simulink model "TosAeb"  (R2021a)
settings: Solver=FixedStepDiscrete  StartTime=0.0  StopTime=inf  FixedStep=0.01  RelTol=1e-3
undefined symbols (not defined in the file or in its folder): ACCModSts, AccSts1, AEB_ObjectMovingOrientation, AEB_ObjectMovingState, CN_BTCActive, CN_CurrentGearR, CN_CurrentGearR_DHT, CN_PTCActive, CN_VDCActive, CN_VSC_EspOff, CN_VSC_FCW_AEB_Error, CN_VSC_FCW_AEB_NoDispay, CN_VSC_FCW_AEB_PerfmDgdtn, CN_VSC_FCW_AEB_TempUnAvlbl, CN_VSC_VehStandstill, CNaccelAvoidMax, CNaccelAvoidMin, CNbrakePedalPress, CNcomfortStrLeftAccelMax, CNcomfortStrLeftAccelMin, CNcomfortStrRightAccelMax, CNcomfortStrRightAccelMin, CNDrvDoorOpen, CNDrvModeEco, CNDrvModeNorm, CNDrvModeOther, CNDrvModeSport, CNDrvSBRreminder, CNNull, CNobjInvalidID, CNPassDoorOpen, CNPassSBRreminder, CNPEBSAccelNegInf, CNPEBSAccelNull, CNPEBSTimeInf, CNPEBSTimeNull, CNSTOPMAX, CNSTOPNULL, CNsubjWidth, CNSwtOFF, CNSwtON, CNTTCMAX, CNTTCNULL, CNVehConfigAEBCycNone, CNVehConfigAEBNone, CNVehConfigAEBPedNone, CNVehConfigFCWNone, CNVehConfigJANone, CollisionSeverity, FALSE, FCWSnvty, JACycObjCalcInfoDefaultParams, JAPedObjCalcInfoDefaultParams, JAVehObjCalcInfoDefaultParams, latCycObjCalcInfoDefaultParams, latPedObjCalcInfoDefaultParams, longObjCalcInfoDefaultParams, ModSts, movingStatus, ObjectType ... (+1112)
libraries: simulink x3031, AEBSFunctionLib x50, Ta_Library x33, AEBSStateMachineLib x23, Mylibrary x12, simulink_extras x7, ObjSelect_lib x3, dspstat3 x2
(part 2 of 102)

== system TosAeb/FcwAebJa_Step_sys/ALG_AEBSystem ==
  TOS_AEB_LONOBJ : Inport  OutDataTypeStr=Bus: Object_Struct  BusOutputAsStruct=on
  TOS_AEB_LATOBJ : Inport  Port=2  OutDataTypeStr=Bus: Object_Struct  PortDimensions=10  Interpolate=off  BusOutputAsStruct=on
  TOS_AEB_JAOBJ : Inport  Port=3  OutDataTypeStr=Bus: Object_Struct  PortDimensions=32  Interpolate=off  BusOutputAsStruct=on
  vxvRefMs : Inport  Port=4  OutDataTypeStr=single  PortDimensions=1
  axvRefMs2 : Inport  Port=5  OutDataTypeStr=single  PortDimensions=1
  ayvRefMs2 : Inport  Port=6  OutDataTypeStr=single  PortDimensions=1
  kapTraj : Inport  Port=7  OutDataTypeStr=single  PortDimensions=1
  psiDtOpt : Inport  Port=8  OutDataTypeStr=single  PortDimensions=1
  ALG_VCAN_MSG : Inport  Port=9  OutDataTypeStr=Bus: VehicleInfo_Struct  PortDimensions=1  BusOutputAsStruct=on
  ALG_AEBController : Subsystem  TreatAsAtomicUnit=on
  ALG_AEBInput : Subsystem  TreatAsAtomicUnit=on
  ALG_AEBOutput : Subsystem  TreatAsAtomicUnit=on
  Signal Conversion{3..7} (5 alike) : SignalConversion  OverrideOpt=off
  AEB_BRK_INFO : Outport  OutDataTypeStr=Bus: AEB_BRK_INFO_BUS  PortDimensions=1
  AEB_DVR_INFO : Outport  Port=2  OutDataTypeStr=Bus: AEB_DVR_INFO_BUS  PortDimensions=1
  AEB_IPHUT_INFO : Outport  Port=3  OutDataTypeStr=Bus: AEB_IPHUT_INFO_BUS  PortDimensions=1
  AEB_EDR_LONOBJ_INFO : Outport  Port=4  OutDataTypeStr=Bus: AEB_EDR_LONOBJ_INFO_BUS  PortDimensions=1
  AEB_EDR_LATPED_INFO : Outport  Port=5  OutDataTypeStr=Bus: AEB_EDR_LATPED_INFO_BUS  PortDimensions=1
  AEB_EDR_LATCYC_INFO : Outport  Port=6  OutDataTypeStr=Bus: AEB_EDR_LATCYC_INFO_BUS  PortDimensions=1
  AEB_EDR_JAPED_INFO : Outport  Port=7  OutDataTypeStr=Bus: AEB_EDR_JAPED_INFO_BUS  PortDimensions=1
  AEB_EDR_JAVEH_INFO : Outport  Port=8  OutDataTypeStr=Bus: AEB_EDR_JAVEH_INFO_BUS  PortDimensions=1
  AEB_FUNC_STS_INFO : Outport  Port=9  OutDataTypeStr=Bus: AEB_FUNC_STS_INFO_BUS
  wires:
  ALG_AEBInput/1(diaManageInfo) -> ALG_AEBController/1(diaManageInfo)
  ALG_AEBInput/2(vehCanInfo) -> ALG_AEBController/2(vehCanInfo)
  ALG_AEBInput/3(vehConfigInfo) -> ALG_AEBController/3(vehConfigInfo)
  ALG_AEBInput/4(longObjInfo) -> ALG_AEBController/4(longObjInfo)
  ALG_AEBInput/5(subjInfo) -> ALG_AEBController/5(subjInfo)
  ALG_AEBInput/6(ACCState) -> ALG_AEBController/6(ACCState)
  ALG_AEBInput/7(LCK_Active) -> ALG_AEBController/7(LCK_Active)
  ALG_AEBInput/8(latObjInfo) -> ALG_AEBController/8(latObjInfo)
  ALG_AEBInput/9(JaObjInfo) -> ALG_AEBController/9(JaObjInfo)
  ALG_AEBController/1(DiaManageInfo) -> ALG_AEBOutput/1(DiaManageInfo)
  ALG_AEBController/2(VehCanInfoOut) -> ALG_AEBOutput/2(VehCanInfo)
  ALG_AEBController/3(vehConfigInfoOut) -> ALG_AEBOutput/3(vehConfigInfoOut)
  ALG_AEBController/4(latPedCalcInfo) -> ALG_AEBOutput/4(latPedCalcInfo)
  ALG_AEBController/5(latCycCalcInfo) -> ALG_AEBOutput/5(latCycCalcInfo)
  ALG_AEBController/6(AEBSFuncOut) -> ALG_AEBOutput/6(AEBSFuncOut)
  ALG_AEBController/7(vehStateCalcInfo) -> ALG_AEBOutput/7(vehStateCalcInfo)
  ALG_AEBController/8(longObjCalcInfo) -> ALG_AEBOutput/8(longObjCalcInfo)
  ALG_AEBController/9(AEBModStsFuncImpArb) -> ALG_AEBOutput/9(AEBModStsFuncImpArb)
  ALG_AEBController/10(AEBModRawSts) -> ALG_AEBOutput/10(AEBModRawSts)
  ALG_AEBController/11(latPedTgtCalcInfo) -> ALG_AEBOutput/11(latPedTgtCalcInfo)
  ALG_AEBController/12(latCycTgtCalcInfo) -> ALG_AEBOutput/12(latCycTgtCalcInfo)
  ALG_AEBController/13(AEBJAPedTgtCalcInfo) -> ALG_AEBOutput/13(AEBJAPedTgtCalcInfo)
  ALG_AEBController/14(JAVehTgtCalcInfo) -> ALG_AEBOutput/14(JAVehTgtCalcInfo)
  ALG_AEBOutput/7(AEB_EDR_JAPED_INFO) -> AEB_EDR_JAPED_INFO/1
  ALG_AEBOutput/8(AEB_EDR_JAVEH_INFO) -> AEB_EDR_JAVEH_INFO/1
  ALG_AEBOutput/9(AEB_FUNC_STS_INFO) -> AEB_FUNC_STS_INFO/1
  ALG_AEBOutput/3(AEB_IPHUT_INFO) -> AEB_IPHUT_INFO/1
  ALG_AEBOutput/6(AEB_EDR_LATCYC_INFO) -> AEB_EDR_LATCYC_INFO/1
  ALG_AEBOutput/4(AEB_EDR_LONOBJ_INFO) -> AEB_EDR_LONOBJ_INFO/1
  ALG_AEBOutput/5(AEB_EDR_LATPED_INFO) -> AEB_EDR_LATPED_INFO/1
  ALG_AEBOutput/2(AEB_DVR_INFO) -> AEB_DVR_INFO/1
  ALG_AEBOutput/1(AEB_BRK_INFO) -> AEB_BRK_INFO/1
  TOS_AEB_JAOBJ/1 -> ALG_AEBInput/3(TOS_AEB_JAOBJ)  "AEB_TOS_AEB_JAOBJ"
  Signal Conversion3/1 -> ALG_AEBInput/4(vxvRefMs)  "AEB_vxvRefMs"
  ayvRefMs2/1 -> Signal Conversion5/1
  Signal Conversion5/1 -> ALG_AEBInput/6(ayvRefMs2)  "AEB_ayvRefMs2"
  TOS_AEB_LONOBJ/1 -> ALG_AEBInput/1(TOS_AEB_LONOBJ)  "AEB_TOS_AEB_LONOBJ"
  TOS_AEB_LATOBJ/1 -> ALG_AEBInput/2(TOS_AEB_LATOBJ)  "AEB_TOS_AEB_LATOBJ"
  Signal Conversion6/1 -> ALG_AEBInput/7(kapTraj)  "AEB_kapTraj"
  kapTraj/1 -> Signal Conversion6/1
  Signal Conversion7/1 -> ALG_AEBInput/8(psiDtOpt)  "AEB_psiDtOpt"
  psiDtOpt/1 -> Signal Conversion7/1
  Signal Conversion4/1 -> ALG_AEBInput/5(axvRefMs2)  "AEB_axvRefMs2"
  axvRefMs2/1 -> Signal Conversion4/1
  vxvRefMs/1 -> Signal Conversion3/1
  ALG_VCAN_MSG/1 -> ALG_AEBInput/9(ALG_VCAN_MSG)
  ALG_AEBController/15(FCWSnvtyALGInfo) -> ALG_AEBOutput/15(FCWSnvtyALGInfo)

== system TosAeb/AEB_Objsel/TA_InfoRebuild/TAObjInfoCalc ==
  Fus_TargetBus : Inport
  Road_Info : Inport  Port=2
  Bus Selector : BusSelector  OutputSignals=Cam_ObsID_u8,Cam_laneLeftPortion,Cam_laneAssociateConf,Cam_laneRightPortion,Cam_laneLeftConf,Cam_laneRightConf,Cam_objIntention,Cam_objIntentionProb,Cam_laneAssign,Cam_ObsPosX_s,Cam_ObsPosY_s,Cam_ObsAccY_s,Cam_ObsAccX_s,Cam_ObsVelY_s,Cam_ObsVelX_s,Cam_ObsID_u8,Cam_ObsValid_u8
  For Each Subsystem : Subsystem  TreatAsAtomicUnit=on
  Signal_Rebuild : Subsystem
  Objrebuild_Struct : Outport
  wires:
  Fus_TargetBus/1 -> Bus Selector/1
  Bus Selector/1 -> For Each Subsystem/1(IDForEach)  "<Cam_ObsID_u8>"
  Bus Selector/15 -> For Each Subsystem/15(Cam_ObsVelX_s)  "<Cam_ObsVelX_s>"
  Bus Selector/14 -> For Each Subsystem/14(Cam_ObsVelY_s)  "<Cam_ObsVelY_s>"
  Bus Selector/13 -> For Each Subsystem/13(Cam_ObsAccX_s)  "<Cam_ObsAccX_s>"
  Bus Selector/12 -> For Each Subsystem/12(Cam_ObsAccY_s)  "<Cam_ObsAccY_s>"
  Bus Selector/11 -> For Each Subsystem/11(Cam_ObsPosY_s)  "<Cam_ObsPosY_s>"
  Bus Selector/10 -> For Each Subsystem/10(Cam_ObsPosX_s)  "<Cam_ObsPosX_s>"
  Bus Selector/9 -> For Each Subsystem/9(Cam_laneAssign)  "<Cam_laneAssign>"
  Bus Selector/8 -> For Each Subsystem/8(Cam_objIntentionProb)  "<Cam_objIntentionProb>"
  Bus Selector/7 -> For Each Subsystem/7(Cam_objIntention)  "<Cam_objIntention>"
  Bus Selector/6 -> For Each Subsystem/6(Cam_laneRightConf)  "<Cam_laneRightConf>"
  Bus Selector/5 -> For Each Subsystem/5(Cam_laneLeftConf)  "<Cam_laneLeftConf>"
  Bus Selector/4 -> For Each Subsystem/4(Cam_laneRightPortion)  "<Cam_laneRightPortion>"
  Bus Selector/3 -> For Each Subsystem/3(Cam_laneAssociateConf)  "<Cam_laneAssociateConf>"
  Bus Selector/2 -> For Each Subsystem/2(Cam_laneLeftPortion)  "<Cam_laneLeftPortion>"
  Road_Info/1 -> For Each Subsystem/18(Road_Info)
  For Each Subsystem/6(ayRel) -> Signal_Rebuild/6(ayRel)
  For Each Subsystem/5(axRel) -> Signal_Rebuild/5(axRel)
  For Each Subsystem/7(axRelStd) -> Signal_Rebuild/7(axRelStd)
  For Each Subsystem/4(vyRelStd) -> Signal_Rebuild/4(vyRelStd)
  For Each Subsystem/8(ayRelStd) -> Signal_Rebuild/8(ayRelStd)
  For Each Subsystem/3(vxRelStd) -> Signal_Rebuild/3(vxRelStd)
  For Each Subsystem/9(azimuthAngle) -> Signal_Rebuild/9(azimuthAngle)
  For Each Subsystem/2(vyRel) -> Signal_Rebuild/2(vyRel)
  For Each Subsystem/10(azimuthAngleStd) -> Signal_Rebuild/10(azimuthAngleStd)
  For Each Subsystem/1(vxRel) -> Signal_Rebuild/1(vxRel)
  For Each Subsystem/11(objLatDist2LaneCenter) -> Signal_Rebuild/11(objLatDist2LaneCenter)
  For Each Subsystem/12(objLatDist2EgoCenter) -> Signal_Rebuild/12(objLatDist2EgoCenter)
  For Each Subsystem/13(objPosition) -> Signal_Rebuild/13(objPosition)
  Signal_Rebuild/1(Objrebuild_Struct) -> Objrebuild_Struct/1
  Bus Selector/16 -> For Each Subsystem/16(Cam_ObsID_u8)  "<Cam_ObsID_u8>"
  Bus Selector/17 -> For Each Subsystem/17(Cam_ObsValid_u8)  "<Cam_ObsValid_u8>"

== system TosAeb/AEB_Objsel/TA_Processor/AEB_ObjSelect ==
  VehSelfData : Inport
  TA_ObjSumInfo : Inport  Port=2
  PathEstimn : Inport  Port=3
  AccTgtIdn : Inport  Port=4
  TargetInfoCheck : Subsystem
  TargetSelect : Subsystem
  Long_ObjSortNum : Outport
  Long_ObjID : Outport  Port=2
  Lat_ObjSortNum : Outport  Port=3
  Lat_ObjID : Outport  Port=4
  Ta_Info : Outport  Port=5
  Long_TTC : Outport  Port=6
  Lat_TTC : Outport  Port=7
  JA_TTC : Outport  Port=8
  wires:
  TargetInfoCheck/11(JA_TTRAssign) -> TargetSelect/11(JA_TTRAssign)
  TA_ObjSumInfo/1 -> TargetInfoCheck/1(TA_ObjSumInfo)
  TargetInfoCheck/1(TA_ObjSumInfo_P) -> TargetSelect/1(TA_ObjInfo)
  VehSelfData/1 -> TargetInfoCheck/2(VehSelfData)
  TargetInfoCheck/2(VehSelfData_P) -> TargetSelect/2(VehSelfData)
  PathEstimn/1 -> TargetInfoCheck/3(PathEstimn)
  AccTgtIdn/1 -> TargetInfoCheck/4(AccTgtIdn)
  TargetSelect/5(Ta_Info) -> Ta_Info/1
  TargetInfoCheck/3(AccTgtIdn_P) -> TargetSelect/3(AccTgtIdn)
  TargetInfoCheck/5(Obj_Coef) -> TargetSelect/5(Obj_Coef)
  TargetInfoCheck/4(Boudingbox_Calculate) -> TargetSelect/4(Boudingbox_Calculate)
  TargetInfoCheck/9(Lat_TTR) -> TargetSelect/9(Lat_TTR)
  TargetInfoCheck/6(TTI) -> TargetSelect/6(TTI)
  TargetSelect/1(Long_ObjSortNum) -> Long_ObjSortNum/1
  TargetInfoCheck/7(LatObj_Valid) -> TargetSelect/7(LatObj_Valid)
  TargetInfoCheck/10(Overlap_Flg) -> TargetSelect/10(Overlap_Flg)
  TargetInfoCheck/8(Lat_ObjIntersect) -> TargetSelect/8(Lat_ObjIntersect)
  TargetSelect/3(Lat_ObjSortNum) -> Lat_ObjSortNum/1
  TargetSelect/2(Long_ObjID) -> Long_ObjID/1
  TargetSelect/7(Lat_TTC) -> Lat_TTC/1
  TargetSelect/4(Lat_ObjID) -> Lat_ObjID/1
  TargetSelect/6(Long_TTC) -> Long_TTC/1
  TargetSelect/8(JA_TTC) -> JA_TTC/1

== system TosAeb/AEB_Objsel/TA_Processor/PreProcessor ==
  VehSelfIn : Inport
  ACC_Infout : Inport  Port=2
  Fus_TargetBus : Inport  Port=3
  ExtractAccTargetIdIfAccActive : Subsystem
  ObjectPrecessor : Subsystem
  VehiclePreProcessor : Subsystem
  VehSelfData : Outport
  TA_ObjSumInfo : Outport  Port=2
  PathEstimn : Outport  Port=3
  AccTgtIdn : Outport  Port=4
  wires:
  Fus_TargetBus/1 -> ObjectPrecessor/2(Fus_TargetBus)
  VehSelfIn/1 -> ObjectPrecessor/1(VehSelfIn), VehiclePreProcessor/1(VehSelfIn)
  ObjectPrecessor/1(TA_ObjInfo) -> TA_ObjSumInfo/1
  ExtractAccTargetIdIfAccActive/1(AccTgtIdn) -> AccTgtIdn/1
  VehiclePreProcessor/2(PathEstimn) -> PathEstimn/1
  ACC_Infout/1 -> ExtractAccTargetIdIfAccActive/1(ACC_Infout)
  VehiclePreProcessor/1(VehSelfData) -> VehSelfData/1

== system TosAeb/AEB_Objsel/TA_Processor/TA_Check ==
  Long_ObjSortNum : Inport
  Long_ObjID : Inport  Port=2
  Lat_ObjSortNum : Inport  Port=3
  Lat_ObjID : Inport  Port=4
  Ta_Info : Inport  Port=5
  Long_TTC : Inport  Port=6
  Lat_TTC : Inport  Port=7
  JA_TTC : Inport  Port=8
  Signal Conversion : SignalConversion  OverrideOpt=off
  TA_LatCheck : Subsystem
  TA_LongCheck : Subsystem
  ObjLong_Bus : Outport
  ObjLat_Bus : Outport  Port=2
  TTC_JA : Outport  Port=3
  wires:
  Lat_ObjSortNum/1 -> TA_LatCheck/1(Lat_ObjSortNum)
  Lat_ObjID/1 -> TA_LatCheck/2(Lat_ObjID)
  Lat_TTC/1 -> TA_LatCheck/3(Lat_TTC)
  Long_ObjSortNum/1 -> TA_LongCheck/1(Long_ObjSortNum)
  Long_ObjID/1 -> TA_LongCheck/2(Long_ObjID)
  Ta_Info/1 -> TA_LongCheck/4(TA_Info)
  Long_TTC/1 -> TA_LongCheck/3(Long_TTC)
  TA_LongCheck/1(ObjLong_Bus) -> ObjLong_Bus/1
  JA_TTC/1 -> Signal Conversion/1
  Signal Conversion/1 -> TTC_JA/1
  TA_LatCheck/1(ObjLat_Bus) -> ObjLat_Bus/1

== system TosAeb/AEB_Objsel/TOS_AEB_Lat_Attributes/If Action Subsystem ==
  Action Port : ActionPort  ActionPortLabel=if(u1 == 0)
  Bus Creator : BusCreator  Inputs=2
  Constant{1..3,10..11,41..44,49..52} (13 alike) : Constant  Value=0  OutDataTypeStr=uint8
  Constant{4,6..9,12..36,45..48,53..56} (38 alike) : Constant  Value=0  OutDataTypeStr=single
  Constant37 : Constant  Value=0  OutDataTypeStr=uint16
  Constant39 : Constant  Value=0  OutDataTypeStr=boolean
  Constant5 : Constant  Value=0  OutDataTypeStr=boolean
  Out1 : Outport
  wires:
  Constant1/1 -> Bus Creator/1  "FusionType"
  Constant2/1 -> Bus Creator/2  "ObjectID"
  Bus Creator/1 -> Out1/1

== system TosAeb/AEB_Objsel/TOS_AEB_Lat_Attributes/If Action Subsystem1 ==
  TOS_AEB_Lat_Index : Inport
  TOS_PP_OFM_SignalVerification : Inport  Port=2
  Action Port : ActionPort  ActionPortLabel=else
  Bus Creator : BusCreator  Inputs=2
  Bus Selector2 : BusSelector  OutputSignals=objID,objType
  Selector : Selector  InputPortWidth=32  IndexOptions=Index vector (port)  Indices=[1 3]  OutputSizes=1
  Selector1 : Selector  InputPortWidth=32  IndexOptions=Index vector (port)  Indices=[1 3]  OutputSizes=1
  Out1 : Outport
  wires:
  TOS_AEB_Lat_Index/1 -> Selector1/2, Selector/2
  TOS_PP_OFM_SignalVerification/1 -> Selector1/1, Selector/1, Bus Selector2/1
  Bus Creator/1 -> Out1/1
  Selector/1 -> Bus Creator/1  "FusionType"
  Selector1/1 -> Bus Creator/2  "ObjectID"

== system TosAeb/ELK_Objsel/ELK_Left_Obj_Delay/Trigger Hold1 ==
  Trigger : Inport
  hold_time : Inport  Port=2
  Chart : Stateflow chart  TreatAsAtomicUnit=on
  Out : Outport
  wires:
  hold_time/1 -> Chart/2(hold_time)
  Trigger/1 -> Chart/1(Trigger)
  Chart/1(Out) -> Out/1

== system TosAeb/ELK_Objsel/ELK_Left_Obj_Delay1/Trigger Hold1 ==
  Trigger : Inport
  hold_time : Inport  Port=2
  Chart : Stateflow chart  TreatAsAtomicUnit=on
  Out : Outport
  wires:
  Chart/1(Out) -> Out/1
  Trigger/1 -> Chart/1(Trigger)
  hold_time/1 -> Chart/2(hold_time)

== system TosAeb/ELK_Objsel/LanesSelect/LineInfo_Process ==
  Leftline_Data : Inport
  Bus Selector : BusSelector  OutputSignals=lineSegNum,lineSegs
  Bus Selector1 : BusSelector  OutputSignals=lineSegValid,lineSegStart,lineSegEnd,lineC0,lineC1,lineC2,lineC3
  Bus Selector2 : BusSelector  OutputSignals=lineSegValid,lineSegStart,lineSegEnd,lineC0,lineC1,lineC2,lineC3
  Bus Selector3 : BusSelector  OutputSignals=lineSegValid,lineSegStart,lineSegEnd,lineC0,lineC1,lineC2,lineC3
  Line_Info : MATLAB Function  TreatAsAtomicUnit=on
  Selector18 : Selector  IndexMode=Zero-based  InputPortWidth=3  IndexOptions=Index vector (dialog)  Indices=[0]  OutputSizes=1
  Selector2 : Selector  IndexMode=Zero-based  InputPortWidth=3  IndexOptions=Index vector (dialog)  Indices=[1]  OutputSizes=1
  Selector3 : Selector  IndexMode=Zero-based  InputPortWidth=3  IndexOptions=Index vector (dialog)  Indices=[2]  OutputSizes=1
  wires:
  Leftline_Data/1 -> Bus Selector/1
  Selector3/1 -> Bus Selector3/1
  Selector18/1 -> Bus Selector1/1
  Selector2/1 -> Bus Selector2/1
  Bus Selector/2 -> Selector3/1, Selector2/1, Selector18/1  "<lineSegs>"
  Bus Selector1/1 -> Line_Info/2(seg1_valid)  "<lineSegValid>"
  Bus Selector1/2 -> Line_Info/3(seg1_Start)  "<lineSegStart>"
  Bus Selector1/3 -> Line_Info/4(seg1_end)  "<lineSegEnd>"
  Bus Selector1/4 -> Line_Info/5(seg1_C0)  "<lineC0>"
  Bus Selector1/5 -> Line_Info/6(seg1_C1)  "<lineC1>"
  Bus Selector1/6 -> Line_Info/7(seg1_C2)  "<lineC2>"
  Bus Selector1/7 -> Line_Info/8(seg1_C3)  "<lineC3>"
  Bus Selector2/1 -> Line_Info/9(seg2_valid)  "<lineSegValid>"
  Bus Selector2/2 -> Line_Info/10(seg2_Start)  "<lineSegStart>"
  Bus Selector2/3 -> Line_Info/11(seg2_end)  "<lineSegEnd>"
  Bus Selector2/4 -> Line_Info/12(seg2_C0)  "<lineC0>"
  Bus Selector2/5 -> Line_Info/13(seg2_C1)  "<lineC1>"
  Bus Selector2/6 -> Line_Info/14(seg2_C2)  "<lineC2>"
  Bus Selector2/7 -> Line_Info/15(seg2_C3)  "<lineC3>"
  Bus Selector3/1 -> Line_Info/16(seg3_valid)  "<lineSegValid>"
  Bus Selector3/2 -> Line_Info/17(seg3_Start)  "<lineSegStart>"
  Bus Selector3/3 -> Line_Info/18(seg3_end)  "<lineSegEnd>"
  Bus Selector3/4 -> Line_Info/19(seg3_C0)  "<lineC0>"
  Bus Selector3/5 -> Line_Info/20(seg3_C1)  "<lineC1>"
  Bus Selector3/6 -> Line_Info/21(seg3_C2)  "<lineC2>"
  Bus Selector3/7 -> Line_Info/22(seg3_C3)  "<lineC3>"
  Bus Selector/1 -> Line_Info/1(seg_num)  "<lineSegNum>"

== system TosAeb/ELK_Objsel/LanesSelect/LineInfo_Process1 ==
  Leftline_Data : Inport
  Bus Selector : BusSelector  OutputSignals=lineSegNum,lineSegs
  Bus Selector1 : BusSelector  OutputSignals=lineSegValid,lineSegStart
  Bus Selector2 : BusSelector  OutputSignals=lineSegValid,lineSegStart
  Bus Selector3 : BusSelector  OutputSignals=lineSegValid,lineSegStart
  Line_Info : MATLAB Function  TreatAsAtomicUnit=on
  Selector18 : Selector  IndexMode=Zero-based  InputPortWidth=3  IndexOptions=Index vector (dialog)  Indices=[0]  OutputSizes=1
  Selector2 : Selector  IndexMode=Zero-based  InputPortWidth=3  IndexOptions=Index vector (dialog)  Indices=[1]  OutputSizes=1
  Selector3 : Selector  IndexMode=Zero-based  InputPortWidth=3  IndexOptions=Index vector (dialog)  Indices=[2]  OutputSizes=1
  Index : Outport
  Valid : Outport  Port=2
  wires:
  Bus Selector/1 -> Line_Info/1(seg_num)  "<lineSegNum>"
  Bus Selector1/2 -> Line_Info/3(seg1_Start)  "<lineSegStart>"
  Bus Selector1/1 -> Line_Info/2(seg1_valid)  "<lineSegValid>"
  Bus Selector/2 -> Selector18/1, Selector2/1, Selector3/1  "<lineSegs>"
  Selector2/1 -> Bus Selector2/1
  Selector18/1 -> Bus Selector1/1
  Selector3/1 -> Bus Selector3/1
  Leftline_Data/1 -> Bus Selector/1
  Line_Info/1(Index) -> Index/1
  Bus Selector2/1 -> Line_Info/4(seg2_valid)  "<lineSegValid>"
  Bus Selector2/2 -> Line_Info/5(seg2_Start)  "<lineSegStart>"
  Bus Selector3/1 -> Line_Info/6(seg3_valid)  "<lineSegValid>"
  Bus Selector3/2 -> Line_Info/7(seg3_Start)  "<lineSegStart>"
  Line_Info/2(Valid) -> Valid/1

== system TosAeb/ELK_Objsel/LanesSelect/LineInfo_Process2 ==
  Rightline_Data : Inport
  Bus Selector : BusSelector  OutputSignals=lineSegNum,lineSegs
  Bus Selector1 : BusSelector  OutputSignals=lineSegValid,lineSegStart
  Bus Selector2 : BusSelector  OutputSignals=lineSegValid,lineSegStart
  Bus Selector3 : BusSelector  OutputSignals=lineSegValid,lineSegStart
  Line_Info : MATLAB Function  TreatAsAtomicUnit=on
  Selector18 : Selector  IndexMode=Zero-based  InputPortWidth=3  IndexOptions=Index vector (dialog)  Indices=[0]  OutputSizes=1
  Selector2 : Selector  IndexMode=Zero-based  InputPortWidth=3  IndexOptions=Index vector (dialog)  Indices=[1]  OutputSizes=1
  Selector3 : Selector  IndexMode=Zero-based  InputPortWidth=3  IndexOptions=Index vector (dialog)  Indices=[2]  OutputSizes=1
  Index : Outport
  Valid : Outport  Port=2
  wires:
  Line_Info/2(Valid) -> Valid/1
  Bus Selector3/2 -> Line_Info/7(seg3_Start)  "<lineSegStart>"
  Bus Selector3/1 -> Line_Info/6(seg3_valid)  "<lineSegValid>"
  Bus Selector2/2 -> Line_Info/5(seg2_Start)  "<lineSegStart>"
  Bus Selector2/1 -> Line_Info/4(seg2_valid)  "<lineSegValid>"
  Line_Info/1(Index) -> Index/1
  Rightline_Data/1 -> Bus Selector/1
  Selector3/1 -> Bus Selector3/1
  Selector18/1 -> Bus Selector1/1
  Selector2/1 -> Bus Selector2/1
  Bus Selector/2 -> Selector3/1, Selector2/1, Selector18/1  "<lineSegs>"
  Bus Selector1/1 -> Line_Info/2(seg1_valid)  "<lineSegValid>"
  Bus Selector1/2 -> Line_Info/3(seg1_Start)  "<lineSegStart>"
  Bus Selector/1 -> Line_Info/1(seg_num)  "<lineSegNum>"

== system TosAeb/ELK_Objsel/Select Object Info1/If Action Subsystem1 ==
  Index : Inport
  Objs_all : Inport  Port=2
  Action Port : ActionPort  ActionPortLabel=else
  Selector : Selector  InputPortWidth=32  IndexOptions=Index vector (port)  Indices=[1 3]  OutputSizes=1
  TargetInfo : Outport
  wires:
  Index/1 -> [Index]
  Objs_all/1 -> [Objs_all]
  [Objs_all] -> Selector/1
  [Index] -> Selector/2
  Selector/1 -> TargetInfo/1

== system TosAeb/ELK_Objsel/Select Object Info1/If Action Subsystem2 ==
  Action Port : ActionPort  ActionPortLabel=if(u1 == 255)
  Bus Creator1 : BusCreator  Inputs=64  OutDataTypeStr=Bus: Object_Struct  NonVirtualBus=on
  Constant : Constant  Value=0  OutDataTypeStr=int64_t
  Constant{1..2,4,8,14..18,20..22,24..27,29..36,38..42,47,50..55,57,62,64..72,74} (48 alike) : Constant  Value=0  OutDataTypeStr=single
  Constant10 : Constant  Value=0  OutDataTypeStr=uint16_t
  Constant11 : Constant  Value=0  OutDataTypeStr=uint16_t
  Constant12 : Constant  Value=0  OutDataTypeStr=bool_t
  Constant{6..7,9,13,19,37,63,73} (8 alike) : Constant  Value=0  OutDataTypeStr=uint8_t
  Constant23 : Constant  Value=100  OutDataTypeStr=single
  Constant28 : Constant  Value=0  OutDataTypeStr=bool_t
  Constant3 : Constant  Value=0  OutDataTypeStr=uint16_t
  Constant5 : Constant  Value=0  OutDataTypeStr=int64_t
  Out : Outport  OutDataTypeStr=Bus:Object_Struct
  wires:
  Constant47/1 -> Bus Creator1/45  "objLengthStd"
  Constant42/1 -> Bus Creator1/44  "objHeight"
  Constant41/1 -> Bus Creator1/42  "objLength"
  Constant40/1 -> Bus Creator1/43  "objWidth"
  Constant39/1 -> Bus Creator1/41  "yawRateStd"
  Constant38/1 -> Bus Creator1/40  "yawRate"
  Constant31/1 -> Bus Creator1/35  "ayAbsStd"
  Constant33/1 -> Bus Creator1/33  "vyAbsStd"
  Constant34/1 -> Bus Creator1/34  "axAbsStd"
  Constant53/1 -> Bus Creator1/36  "yawAngle"
  Constant55/1 -> Bus Creator1/38  "azimuthAngle"
  Constant54/1 -> Bus Creator1/37  "yawAngleStd"
  Constant57/1 -> Bus Creator1/39  "azimuthAngleStd"
  Constant52/1 -> Bus Creator1/32  "vxAbsStd"
  Constant51/1 -> Bus Creator1/31  "ayAbs"
  Constant50/1 -> Bus Creator1/30  "axAbs"
  Constant71/1 -> Bus Creator1/28  "vxAbs"
  Constant72/1 -> Bus Creator1/29  "vyAbs"
  Constant68/1 -> Bus Creator1/27  "ayRelStd"
  Constant27/1 -> Bus Creator1/23  "ayRel"
  Constant32/1 -> Bus Creator1/26  "axRelStd"
  Constant30/1 -> Bus Creator1/25  "vyRelStd"
  Constant29/1 -> Bus Creator1/24  "vxRelStd"
  Constant67/1 -> Bus Creator1/22  "axRel"
  Constant66/1 -> Bus Creator1/21  "vyRel"
  Constant74/1 -> Bus Creator1/20  "vxRel"
  Constant73/1 -> Bus Creator1/19  "surfacePos"
  Constant62/1 -> Bus Creator1/15  "dyRef"
  Constant64/1 -> Bus Creator1/18  "dyRefSurface"
  Constant65/1 -> Bus Creator1/17  "dxRefSurface"
  Constant63/1 -> Bus Creator1/16  "refPosition"
  Bus Creator1/1 -> Out/1
  Constant26/1 -> Bus Creator1/14  "dxRef"
  Constant25/1 -> Bus Creator1/13  "dyCenterStd"
  Constant8/1 -> Bus Creator1/12  "dyCenter"
  Constant24/1 -> Bus Creator1/11  "dxCenterStd"
  Constant21/1 -> Bus Creator1/10  "dxCenter"
  Constant20/1 -> Bus Creator1/9  "objTypeProb"
  Constant6/1 -> Bus Creator1/8  "objType"
  Constant5/1 -> Bus Creator1/7  "fusionType"
  Constant4/1 -> Bus Creator1/6  "objLifeCycle"
  Constant70/1 -> Bus Creator1/5  "objIsobsProb"
  Constant69/1 -> Bus Creator1/4  "objExitProb"
  Constant28/1 -> Bus Creator1/3  "objValid"
  Constant3/1 -> Bus Creator1/2  "objID"
  Constant/1 -> Bus Creator1/1  "objTimeStamp"
  Constant1/1 -> Bus Creator1/46  "objWidthStd"
  Constant2/1 -> Bus Creator1/47  "objHeightStd"
  Constant7/1 -> Bus Creator1/48  "movingStatus"
  Constant9/1 -> Bus Creator1/49  "movingDirection"
  Constant10/1 -> Bus Creator1/50  "lightStatus"
  Constant11/1 -> Bus Creator1/51  "doorStatus"
  Constant12/1 -> Bus Creator1/52  "trailerStatus"
  Constant13/1 -> Bus Creator1/53  "laneAssign"
  Constant14/1 -> Bus Creator1/54  "laneAssociateConf"
  Constant15/1 -> Bus Creator1/55  "laneLeftPortion"
  Constant16/1 -> Bus Creator1/56  "laneRightPortion"
  Constant17/1 -> Bus Creator1/57  "laneLeftConf"
  Constant18/1 -> Bus Creator1/58  "laneRightConf"
  Constant19/1 -> Bus Creator1/59  "objIntention"
  Constant22/1 -> Bus Creator1/60  "objIntentionProb"
  Constant23/1 -> Bus Creator1/61  "objTTC"
  Constant35/1 -> Bus Creator1/62  "objLatDist2LaneCenter"
  Constant36/1 -> Bus Creator1/63  "objLatDist2EgoCenter"
  Constant37/1 -> Bus Creator1/64  "objPosition"
